simulink library "lib_all"  (Simulink 7.6)
settings: Solver=ode45  SolverMode=SingleTasking  StartTime=0.0  StopTime=10.0  RelTol=1e-3
values from neighbouring files (symbols this model uses):
  map_lib_03 = [6.8679 7.0159 7.4571 8.1849 9.1892 10.456 11.97 13.71 15.655 17.781 20.063 22.474 ... (803 elements, 73x11)]    [scr_simdata.mat]    (also in scr_simdata_all.mat)
  map_lib_06 = [2.5784 2.6727 2.9588 3.4453 4.1444 5.068 6.226 7.6242 9.2634 11.139 13.24 15.552 ... (803 elements, 73x11)]    [scr_simdata.mat]    (also in scr_simdata_all.mat)
  map_lib_09 = [0.0025784 0.0026727 0.0029588 0.0034453 0.0041444 0.005068 0.006226 0.0076242 0.0092634 0.011139 0.01324 0.015552 ... (803 elements, 73x11)]    [scr_simdata.mat]    (also in scr_simdata_all.mat)
  map_lib_10 = [2.74 2.75 2.76 2.77 2.78 2.79 2.8 2.81 2.82 2.83 2.84 2.85 ... (81 elements, 1x81)]    [scr_simdata.mat]    (also in scr_simdata_all.mat)
  map_lib_11 = [1.1983 1.2027 1.207 1.2113 1.2156 1.2198 1.2241 1.2283 1.2326 1.2368 1.241 1.2451 ... (81 elements, 1x81)]    [scr_simdata.mat]    (also in scr_simdata_all.mat)
  map_lib_12 = [2.74 2.75 2.76 2.77 2.78 2.79 2.8 2.81 2.82 2.83 2.84 2.85 ... (81 elements, 1x81)]    [scr_simdata.mat]    (also in scr_simdata_all.mat)
  map_lib_13 = [1.74 1.75 1.76 1.77 1.78 1.79 1.8 1.81 1.82 1.83 1.84 1.85 ... (81 elements, 1x81)]    [scr_simdata.mat]    (also in scr_simdata_all.mat)
  map_lib_16 = [0.00048828125 1.6159 1.8962 2.0821 2.2251 2.3426 2.4433 2.5318 2.611 2.683 2.749 2.8101 ... (1419 elements, 129x11)]    [scr_simdata.mat]    (also in scr_simdata_all.mat)
  map_mr2_01 = [0 800 1600 2400 3200 4000]    [scr_simdata.mat]    (also in scr_simdata_all.mat)
  map_mr2_02 = [1.32 1.32 1.33 1.34 1.36 1.38]    [scr_simdata.mat]    (also in scr_simdata_all.mat)
  map_mr2_03 = [0 800 1600 2400 3200 4000]    [scr_simdata.mat]    (also in scr_simdata_all.mat)
  map_mr2_04 = [1.32 1.32 1.33 1.34 1.36 1.38]    [scr_simdata.mat]    (also in scr_simdata_all.mat)
  map_mr2_05 = [0 800 1600 2400 3200 4000]    [scr_simdata.mat]    (also in scr_simdata_all.mat)
  map_mr2_06 = [1.32 1.32 1.33 1.34 1.36 1.38]    [scr_simdata.mat]    (also in scr_simdata_all.mat)
  Parameter1 = 6    [scr_simdata.mat]    (also in scr_simdata_all.mat)
  Parameter101 = 30    [scr_simdata.mat]    (also in scr_simdata_all.mat)
  Parameter102 = 12    [scr_simdata.mat]    (also in scr_simdata_all.mat)
  Parameter103 = -240    [scr_simdata.mat]    (also in scr_simdata_all.mat)
  Parameter108 = 48    [scr_simdata.mat]    (also in scr_simdata_all.mat)
  Parameter120 = 16    [scr_simdata.mat]    (also in scr_simdata_all.mat)
  Parameter121 = 87    [scr_simdata.mat]    (also in scr_simdata_all.mat)
  Parameter123 = 78    [scr_simdata.mat]    (also in scr_simdata_all.mat)
  Parameter124 = 60    [scr_simdata.mat]    (also in scr_simdata_all.mat)
  Parameter125 = -480    [scr_simdata.mat]    (also in scr_simdata_all.mat)
  Parameter130 = 96    [scr_simdata.mat]    (also in scr_simdata_all.mat)
  Parameter131 = 3    [scr_simdata.mat]    (also in scr_simdata_all.mat)
  Parameter144 = 3    [scr_simdata.mat]    (also in scr_simdata_all.mat)
  Parameter18 = 128    [scr_simdata.mat]    (also in scr_simdata_all.mat)
  Parameter19 = 16    [scr_simdata.mat]    (also in scr_simdata_all.mat)
  Parameter2 = 12    [scr_simdata.mat]    (also in scr_simdata_all.mat)
  Parameter20 = 7    [scr_simdata.mat]    (also in scr_simdata_all.mat)
  Parameter21 = 4    [scr_simdata.mat]    (also in scr_simdata_all.mat)
  Parameter3 = 18    [scr_simdata.mat]    (also in scr_simdata_all.mat)
  Parameter32 = 1    [scr_simdata.mat]    (also in scr_simdata_all.mat)
  Parameter34 = 24    [scr_simdata.mat]    (also in scr_simdata_all.mat)
  Parameter4 = 138    [scr_simdata.mat]    (also in scr_simdata_all.mat)
  Parameter42 = 48    [scr_simdata.mat]    (also in scr_simdata_all.mat)
  Parameter5 = 135    [scr_simdata.mat]    (also in scr_simdata_all.mat)
  Parameter55 = 0    [scr_simdata.mat]    (also in scr_simdata_all.mat)
  Parameter56 = 0    [scr_simdata.mat]    (also in scr_simdata_all.mat)
  Parameter57 = 1    [scr_simdata.mat]    (also in scr_simdata_all.mat)
  Parameter58 = 1    [scr_simdata.mat]    (also in scr_simdata_all.mat)
  Parameter59 = 2    [scr_simdata.mat]    (also in scr_simdata_all.mat)
  Parameter6 = 132    [scr_simdata.mat]    (also in scr_simdata_all.mat)
  Parameter60 = 2    [scr_simdata.mat]    (also in scr_simdata_all.mat)
  Parameter63 = 144    [scr_simdata.mat]    (also in scr_simdata_all.mat)
  Parameter7 = 126    [scr_simdata.mat]    (also in scr_simdata_all.mat)
  Parameter76 = 64    [scr_simdata.mat]    (also in scr_simdata_all.mat)
  Parameter77 = 135    [scr_simdata.mat]    (also in scr_simdata_all.mat)
  Parameter79 = 126    [scr_simdata.mat]    (also in scr_simdata_all.mat)
  Parameter80 = 108    [scr_simdata.mat]    (also in scr_simdata_all.mat)
  Parameter81 = 0    [scr_simdata.mat]    (also in scr_simdata_all.mat)
  Parameter86 = 0    [scr_simdata.mat]    (also in scr_simdata_all.mat)
  Parameter98 = 112    [scr_simdata.mat]    (also in scr_simdata_all.mat)
  Parameter99 = 39    [scr_simdata.mat]    (also in scr_simdata_all.mat)
undefined symbols (not defined in the file or in its folder): cylvolkp_hih_map2h, cylvolkp_map2h, pk1k_map2h
libraries: simulink x2
(part 19 of 36)

== system lib_all/SubSystem1593/SubSystem1648/SubSystem1650/SubSystem1651/SubSystem1653 ==
  note: ***編集禁止***
  In1 : Inport
  In2 : Inport  Port=2
  RO1 : RelationalOperator  Operator===  InputSameDT=on
  single1 : DataTypeConversion  OutDataTypeStr=single  SaturateOnIntegerOverflow=on  RndMeth=Zero
  single2 : DataTypeConversion  OutDataTypeStr=single  SaturateOnIntegerOverflow=on  RndMeth=Zero
  Out1 : Outport
  wires:
  RO1/1 -> Out1/1
  In2/1 -> single2/1
  In1/1 -> single1/1
  single1/1 -> RO1/1
  single2/1 -> RO1/2

== system lib_all/SubSystem1593/SubSystem1648/SubSystem1650/SubSystem1651/SubSystem1654 ==
  Block4415 : Inport
  Block4416 : Inport  Port=2
  Block4417 : Inport  Port=3
  Block4418 : Sum  OutDataTypeStr=double  RndMeth=Zero  SaturateOnIntegerOverflow=on  InputSameDT=on
  Block4419 : Switch  Threshold=0.5  RndMeth=Zero  SaturateOnIntegerOverflow=on  InputSameDT=on
  SubSystem1655 : Subsystem
  Block4420 : Outport
  wires:
  Block4415/1 -> Block4418/1
  Block4419/1 -> Block4420/1
  Block4418/1 -> Block4419/1
  SubSystem1655/1(Out1) -> Block4419/2
  Block4417/1 -> SubSystem1655/2(In2)
  Block4416/1 -> Block4418/2, Block4419/3, SubSystem1655/1(In1)

== system lib_all/SubSystem1593/SubSystem1648/SubSystem1650/SubSystem1656/SubSystem1657 ==
  note: ***編集禁止***
  In1 : Inport
  In2 : Inport  Port=2
  LO1 : Logic
  RO1 : RelationalOperator  Operator=<  InputSameDT=on
  RO2 : RelationalOperator  Operator=~=  InputSameDT=on
  single1 : DataTypeConversion  OutDataTypeStr=single  SaturateOnIntegerOverflow=on  RndMeth=Zero
  single2 : DataTypeConversion  OutDataTypeStr=single  SaturateOnIntegerOverflow=on  RndMeth=Zero
  Out1 : Outport
  wires:
  In2/1 -> single2/1
  In1/1 -> single1/1
  single1/1 -> RO1/1, RO2/1
  single2/1 -> RO1/2, RO2/2
  RO1/1 -> LO1/1
  RO2/1 -> LO1/2
  LO1/1 -> Out1/1

== system lib_all/SubSystem1662/SubSystem1664/SubSystem1665/SubSystem1668/SubSystem1669 ==
  note: ***編集禁止***
  In1 : Inport
  In2 : Inport  Port=2
  LO1 : Logic
  RO1 : RelationalOperator  Operator=<  InputSameDT=on
  RO2 : RelationalOperator  Operator=~=  InputSameDT=on
  single1 : DataTypeConversion  OutDataTypeStr=single  SaturateOnIntegerOverflow=on  RndMeth=Zero
  single2 : DataTypeConversion  OutDataTypeStr=single  SaturateOnIntegerOverflow=on  RndMeth=Zero
  Out1 : Outport
  wires:
  In2/1 -> single2/1
  In1/1 -> single1/1
  single1/1 -> RO1/1, RO2/1
  single2/1 -> RO1/2, RO2/2
  RO1/1 -> LO1/1
  RO2/1 -> LO1/2
  LO1/1 -> Out1/1

== system lib_all/SubSystem1674/SubSystem1687/SubSystem1690/SubSystem1691/SubSystem1692 ==
  note: ***編集禁止***
  In1 : Inport
  In2 : Inport  Port=2
  RO1 : RelationalOperator  Operator===  InputSameDT=on
  single1 : DataTypeConversion  OutDataTypeStr=single  SaturateOnIntegerOverflow=on  RndMeth=Zero
  single2 : DataTypeConversion  OutDataTypeStr=single  SaturateOnIntegerOverflow=on  RndMeth=Zero
  Out1 : Outport
  wires:
  single2/1 -> RO1/2
  single1/1 -> RO1/1
  In1/1 -> single1/1
  In2/1 -> single2/1
  RO1/1 -> Out1/1

== system lib_all/SubSystem1674/SubSystem1687/SubSystem1690/SubSystem1691/SubSystem1693 ==
  note: ***編集禁止***
  In1 : Inport
  In2 : Inport  Port=2
  RO1 : RelationalOperator  Operator===  InputSameDT=on
  single1 : DataTypeConversion  OutDataTypeStr=single  SaturateOnIntegerOverflow=on  RndMeth=Zero
  single2 : DataTypeConversion  OutDataTypeStr=single  SaturateOnIntegerOverflow=on  RndMeth=Zero
  Out1 : Outport
  wires:
  single2/1 -> RO1/2
  single1/1 -> RO1/1
  In1/1 -> single1/1
  In2/1 -> single2/1
  RO1/1 -> Out1/1

== system lib_all/SubSystem1674/SubSystem1687/SubSystem1690/SubSystem1691/SubSystem1694 ==
  Block4594 : Inport
  Block4595 : Inport  Port=2
  Block4596 : Inport  Port=3
  Block4597 : Sum  OutDataTypeStr=double  RndMeth=Zero  SaturateOnIntegerOverflow=on  InputSameDT=on
  Block4598 : Switch  Threshold=0.5  RndMeth=Zero  SaturateOnIntegerOverflow=on  InputSameDT=on
  SubSystem1695 : Subsystem
  Block4599 : Outport
  wires:
  Block4595/1 -> SubSystem1695/1(In1), Block4598/3, Block4597/2
  Block4596/1 -> SubSystem1695/2(In2)
  SubSystem1695/1(Out1) -> Block4598/2
  Block4597/1 -> Block4598/1
  Block4598/1 -> Block4599/1
  Block4594/1 -> Block4597/1

== system lib_all/SubSystem1674/SubSystem1687/SubSystem1690/SubSystem1696/SubSystem1697 ==
  note: ***編集禁止***
  In1 : Inport
  In2 : Inport  Port=2
  LO1 : Logic
  RO1 : RelationalOperator  Operator=<  InputSameDT=on
  RO2 : RelationalOperator  Operator=~=  InputSameDT=on
  single1 : DataTypeConversion  OutDataTypeStr=single  SaturateOnIntegerOverflow=on  RndMeth=Zero
  single2 : DataTypeConversion  OutDataTypeStr=single  SaturateOnIntegerOverflow=on  RndMeth=Zero
  Out1 : Outport
  wires:
  LO1/1 -> Out1/1
  RO2/1 -> LO1/2
  RO1/1 -> LO1/1
  single2/1 -> RO2/2, RO1/2
  single1/1 -> RO2/1, RO1/1
  In1/1 -> single1/1
  In2/1 -> single2/1

== system lib_all/SubSystem1674/SubSystem1701/SubSystem1703/SubSystem1704/Product ==
  note: ***編集禁止***
  mult : Inport
  div : Inport  Port=2
  init : Inport  Port=3
  Constant1 : Constant  OutDataTypeStr=Inherit: Inherit via back propagation
  Constant2 : Constant  Value=0  OutDataTypeStr=Inherit: Inherit via back propagation
  Product : Product  Inputs=*/  SaturateOnIntegerOverflow=on  RndMeth=Zero  InputSameDT=on  OutDataTypeStr=Inherit: Same as first input
  Relational Operator : RelationalOperator  Operator=~=  InputSameDT=on
  Switch : Switch  Threshold=0.5  SaturateOnIntegerOverflow=on  InputSameDT=on
  Switch1 : Switch  Threshold=0.5  SaturateOnIntegerOverflow=on  InputSameDT=on
  Out : Outport
  wires:
  mult/1 -> Product/1
  Product/1 -> Switch/1
  Relational Operator/1 -> Switch/2, Switch1/2
  init/1 -> Switch/3
  Switch/1 -> Out/1
  div/1 -> Switch1/1, Relational Operator/1
  Switch1/1 -> Product/2
  Constant2/1 -> Relational Operator/2
  Constant1/1 -> Switch1/3

== system lib_all/SubSystem1674/SubSystem1701/SubSystem1703/SubSystem1706/SubSystem1707 ==
  note: ***編集禁止***
  In1 : Inport
  In2 : Inport  Port=2
  RO1 : RelationalOperator  Operator=>  InputSameDT=on
  single1 : DataTypeConversion  OutDataTypeStr=single  SaturateOnIntegerOverflow=on  RndMeth=Zero
  single2 : DataTypeConversion  OutDataTypeStr=single  SaturateOnIntegerOverflow=on  RndMeth=Zero
  Out1 : Outport
  wires:
  single2/1 -> RO1/2
  single1/1 -> RO1/1
  In1/1 -> single1/1
  In2/1 -> single2/1
  RO1/1 -> Out1/1

== system lib_all/SubSystem1674/SubSystem1701/SubSystem1703/SubSystem1706/SubSystem1708 ==
  note: ***編集禁止***
  In1 : Inport
  In2 : Inport  Port=2
  LO1 : Logic
  RO1 : RelationalOperator  Operator=<  InputSameDT=on
  RO2 : RelationalOperator  Operator=~=  InputSameDT=on
  single1 : DataTypeConversion  OutDataTypeStr=single  SaturateOnIntegerOverflow=on  RndMeth=Zero
  single2 : DataTypeConversion  OutDataTypeStr=single  SaturateOnIntegerOverflow=on  RndMeth=Zero
  Out1 : Outport
  wires:
  LO1/1 -> Out1/1
  RO2/1 -> LO1/2
  RO1/1 -> LO1/1
  single2/1 -> RO2/2, RO1/2
  single1/1 -> RO2/1, RO1/1
  In1/1 -> single1/1
  In2/1 -> single2/1

== system lib_all/SubSystem1674/SubSystem1701/SubSystem1709/SubSystem1713/SubSystem1714 ==
  Block4682 : Inport
  Block4683 : Inport  Port=2
  Block4684 : MultiPortSwitch  zeroidx=off  RndMeth=Zero  SaturateOnIntegerOverflow=on  InputSameDT=on
  SubSystem1715 : Subsystem  TreatAsAtomicUnit=on  mask(***ATC.DSRVEC***){bd_version=1.01.A, r0=DSM18, r1=-1, r2=, r3=, r4=, r5=, r6=, r7=, r8=, r9=, c0=, c1=, c2=, c3=, c4=, c5=, c6=, c7=, c8=, c9=}
      mask prompts: bd_version "Version"
      mask help: これはAutoCからのブロックです．編集禁止．
      settings: OpenFcn=warndlg('変更禁止!');
      mask Help: web(ui_blockhelpmng(gcb));
      mask Display: disp('ATC.DSRVEC')
  SubSystem1716 : Subsystem  TreatAsAtomicUnit=on  mask(***ATC.DSRVEC***){bd_version=1.01.A, r0=DSM8, r1=-1, r2=, r3=, r4=, r5=, r6=, r7=, r8=, r9=, c0=, c1=, c2=, c3=, c4=, c5=, c6=, c7=, c8=, c9=}
      mask prompts: bd_version "Version"
      mask help: これはAutoCからのブロックです．編集禁止．
      settings: OpenFcn=warndlg('変更禁止!');
      mask Help: web(ui_blockhelpmng(gcb));
      mask Display: disp('ATC.DSRVEC')
  SubSystem1717 : Subsystem  TreatAsAtomicUnit=on  mask(***ATC.DSRVEC***){bd_version=1.01.A, r0=DSM11, r1=-1, r2=, r3=, r4=, r5=, r6=, r7=, r8=, r9=, c0=, c1=, c2=, c3=, c4=, c5=, c6=, c7=, c8=, c9=}
      mask prompts: bd_version "Version"
      mask help: これはAutoCからのブロックです．編集禁止．
      settings: OpenFcn=warndlg('変更禁止!');
      mask Help: web(ui_blockhelpmng(gcb));
      mask Display: disp('ATC.DSRVEC')
  Block4685 : Outport
  wires:
  Block4683/1 -> SubSystem1717/1(element), SubSystem1716/1(element), SubSystem1715/1(element)
  SubSystem1717/1(Output) -> Block4684/4
  SubSystem1716/1(Output) -> Block4684/3
  SubSystem1715/1(Output) -> Block4684/2
  Block4684/1 -> Block4685/1
  Block4682/1 -> Block4684/1

== system lib_all/SubSystem1674/SubSystem1701/SubSystem1709/SubSystem1713/SubSystem1718 ==
  Block4686 : Inport
  Block4687 : Inport  Port=2
  Block4688 : MultiPortSwitch  zeroidx=off  RndMeth=Zero  SaturateOnIntegerOverflow=on  InputSameDT=on
  SubSystem1719 : Subsystem  TreatAsAtomicUnit=on  mask(***ATC.DSRVEC***){bd_version=1.01.A, r0=DSM18, r1=-1, r2=, r3=, r4=, r5=, r6=, r7=, r8=, r9=, c0=, c1=, c2=, c3=, c4=, c5=, c6=, c7=, c8=, c9=}
      mask prompts: bd_version "Version"
      mask help: これはAutoCからのブロックです．編集禁止．
      settings: OpenFcn=warndlg('変更禁止!');
      mask Help: web(ui_blockhelpmng(gcb));
      mask Display: disp('ATC.DSRVEC')
  SubSystem1720 : Subsystem  TreatAsAtomicUnit=on  mask(***ATC.DSRVEC***){bd_version=1.01.A, r0=DSM8, r1=-1, r2=, r3=, r4=, r5=, r6=, r7=, r8=, r9=, c0=, c1=, c2=, c3=, c4=, c5=, c6=, c7=, c8=, c9=}
      mask prompts: bd_version "Version"
      mask help: これはAutoCからのブロックです．編集禁止．
      settings: OpenFcn=warndlg('変更禁止!');
      mask Help: web(ui_blockhelpmng(gcb));
      mask Display: disp('ATC.DSRVEC')
  SubSystem1721 : Subsystem  TreatAsAtomicUnit=on  mask(***ATC.DSRVEC***){bd_version=1.01.A, r0=DSM11, r1=-1, r2=, r3=, r4=, r5=, r6=, r7=, r8=, r9=, c0=, c1=, c2=, c3=, c4=, c5=, c6=, c7=, c8=, c9=}
      mask prompts: bd_version "Version"
      mask help: これはAutoCからのブロックです．編集禁止．
      settings: OpenFcn=warndlg('変更禁止!');
      mask Help: web(ui_blockhelpmng(gcb));
      mask Display: disp('ATC.DSRVEC')
  Block4689 : Outport
  wires:
  Block4687/1 -> SubSystem1721/1(element), SubSystem1720/1(element), SubSystem1719/1(element)
  SubSystem1721/1(Output) -> Block4688/4
  SubSystem1720/1(Output) -> Block4688/3
  SubSystem1719/1(Output) -> Block4688/2
  Block4688/1 -> Block4689/1
  Block4686/1 -> Block4688/1

== system lib_all/SubSystem1674/SubSystem1701/SubSystem1709/SubSystem1722/SubSystem1723 ==
  note: ***編集禁止***
  element : Inport
  Inside_DSR : DataStoreRead  DataStoreName=DSM4
  Selector : Selector  IndexOptions=Index vector (port)  Indices=[1 3]  OutputSizes=1
  Output : Outport
  wires:
  Inside_DSR/1 -> Selector/1
  element/1 -> Selector/2
  Selector/1 -> Output/1

== system lib_all/SubSystem1674/SubSystem1701/SubSystem1709/SubSystem1722/SubSystem1724 ==
  Block4697 : Inport
  Block4698 : Inport  Port=2
  Block4699 : MultiPortSwitch  zeroidx=off  RndMeth=Zero  SaturateOnIntegerOverflow=on  InputSameDT=on
  SubSystem1725 : Subsystem  TreatAsAtomicUnit=on  mask(***ATC.DSRVEC***){bd_version=1.01.A, r0=DSM18, r1=-1, r2=, r3=, r4=, r5=, r6=, r7=, r8=, r9=, c0=, c1=, c2=, c3=, c4=, c5=, c6=, c7=, c8=, c9=}
      mask prompts: bd_version "Version"
      mask help: これはAutoCからのブロックです．編集禁止．
      settings: OpenFcn=warndlg('変更禁止!');
      mask Help: web(ui_blockhelpmng(gcb));
      mask Display: disp('ATC.DSRVEC')
  SubSystem1726 : Subsystem  TreatAsAtomicUnit=on  mask(***ATC.DSRVEC***){bd_version=1.01.A, r0=DSM8, r1=-1, r2=, r3=, r4=, r5=, r6=, r7=, r8=, r9=, c0=, c1=, c2=, c3=, c4=, c5=, c6=, c7=, c8=, c9=}
      mask prompts: bd_version "Version"
      mask help: これはAutoCからのブロックです．編集禁止．
      settings: OpenFcn=warndlg('変更禁止!');
      mask Help: web(ui_blockhelpmng(gcb));
      mask Display: disp('ATC.DSRVEC')
  SubSystem1727 : Subsystem  TreatAsAtomicUnit=on  mask(***ATC.DSRVEC***){bd_version=1.01.A, r0=DSM11, r1=-1, r2=, r3=, r4=, r5=, r6=, r7=, r8=, r9=, c0=, c1=, c2=, c3=, c4=, c5=, c6=, c7=, c8=, c9=}
      mask prompts: bd_version "Version"
      mask help: これはAutoCからのブロックです．編集禁止．
      settings: OpenFcn=warndlg('変更禁止!');
      mask Help: web(ui_blockhelpmng(gcb));
      mask Display: disp('ATC.DSRVEC')
  Block4700 : Outport
  wires:
  Block4698/1 -> SubSystem1727/1(element), SubSystem1726/1(element), SubSystem1725/1(element)
  SubSystem1727/1(Output) -> Block4699/4
  SubSystem1726/1(Output) -> Block4699/3
  SubSystem1725/1(Output) -> Block4699/2
  Block4699/1 -> Block4700/1
  Block4697/1 -> Block4699/1

== system lib_all/SubSystem1674/SubSystem1701/SubSystem1709/SubSystem1722/SubSystem1728 ==
  Block4701 : Inport
  Block4702 : Inport  Port=2
  Block4703 : MultiPortSwitch  zeroidx=off  RndMeth=Zero  SaturateOnIntegerOverflow=on  InputSameDT=on
  SubSystem1729 : Subsystem  TreatAsAtomicUnit=on  mask(***ATC.DSRVEC***){bd_version=1.01.A, r0=DSM18, r1=-1, r2=, r3=, r4=, r5=, r6=, r7=, r8=, r9=, c0=, c1=, c2=, c3=, c4=, c5=, c6=, c7=, c8=, c9=}
      mask prompts: bd_version "Version"
      mask help: これはAutoCからのブロックです．編集禁止．
      settings: OpenFcn=warndlg('変更禁止!');
      mask Help: web(ui_blockhelpmng(gcb));
      mask Display: disp('ATC.DSRVEC')
  SubSystem1730 : Subsystem  TreatAsAtomicUnit=on  mask(***ATC.DSRVEC***){bd_version=1.01.A, r0=DSM8, r1=-1, r2=, r3=, r4=, r5=, r6=, r7=, r8=, r9=, c0=, c1=, c2=, c3=, c4=, c5=, c6=, c7=, c8=, c9=}
      mask prompts: bd_version "Version"
      mask help: これはAutoCからのブロックです．編集禁止．
      settings: OpenFcn=warndlg('変更禁止!');
      mask Help: web(ui_blockhelpmng(gcb));
      mask Display: disp('ATC.DSRVEC')
  SubSystem1731 : Subsystem  TreatAsAtomicUnit=on  mask(***ATC.DSRVEC***){bd_version=1.01.A, r0=DSM11, r1=-1, r2=, r3=, r4=, r5=, r6=, r7=, r8=, r9=, c0=, c1=, c2=, c3=, c4=, c5=, c6=, c7=, c8=, c9=}
      mask prompts: bd_version "Version"
      mask help: これはAutoCからのブロックです．編集禁止．
      settings: OpenFcn=warndlg('変更禁止!');
      mask Help: web(ui_blockhelpmng(gcb));
      mask Display: disp('ATC.DSRVEC')
  Block4704 : Outport
  wires:
  Block4702/1 -> SubSystem1731/1(element), SubSystem1730/1(element), SubSystem1729/1(element)
  SubSystem1731/1(Output) -> Block4703/4
  SubSystem1730/1(Output) -> Block4703/3
  SubSystem1729/1(Output) -> Block4703/2
  Block4703/1 -> Block4704/1
  Block4701/1 -> Block4703/1

== system lib_all/SubSystem1674/SubSystem1701/SubSystem1709/SubSystem1732/SubSystem1733 ==
  note: ***編集禁止***
  element : Inport
  Inside_DSR : DataStoreRead  DataStoreName=DSM2
  Selector : Selector  IndexOptions=Index vector (port)  Indices=[1 3]  OutputSizes=1
  Output : Outport
  wires:
  Inside_DSR/1 -> Selector/1
  element/1 -> Selector/2
  Selector/1 -> Output/1

== system lib_all/SubSystem1674/SubSystem1701/SubSystem1709/SubSystem1732/SubSystem1734 ==
  Block4712 : Inport
  Block4713 : Inport  Port=2
  Block4714 : MultiPortSwitch  zeroidx=off  RndMeth=Zero  SaturateOnIntegerOverflow=on  InputSameDT=on
  SubSystem1735 : Subsystem  TreatAsAtomicUnit=on  mask(***ATC.DSRVEC***){bd_version=1.01.A, r0=DSM18, r1=-1, r2=, r3=, r4=, r5=, r6=, r7=, r8=, r9=, c0=, c1=, c2=, c3=, c4=, c5=, c6=, c7=, c8=, c9=}
      mask prompts: bd_version "Version"
      mask help: これはAutoCからのブロックです．編集禁止．
      settings: OpenFcn=warndlg('変更禁止!');
      mask Help: web(ui_blockhelpmng(gcb));
      mask Display: disp('ATC.DSRVEC')
  SubSystem1736 : Subsystem  TreatAsAtomicUnit=on  mask(***ATC.DSRVEC***){bd_version=1.01.A, r0=DSM8, r1=-1, r2=, r3=, r4=, r5=, r6=, r7=, r8=, r9=, c0=, c1=, c2=, c3=, c4=, c5=, c6=, c7=, c8=, c9=}
      mask prompts: bd_version "Version"
      mask help: これはAutoCからのブロックです．編集禁止．
      settings: OpenFcn=warndlg('変更禁止!');
      mask Help: web(ui_blockhelpmng(gcb));
      mask Display: disp('ATC.DSRVEC')
  SubSystem1737 : Subsystem  TreatAsAtomicUnit=on  mask(***ATC.DSRVEC***){bd_version=1.01.A, r0=DSM11, r1=-1, r2=, r3=, r4=, r5=, r6=, r7=, r8=, r9=, c0=, c1=, c2=, c3=, c4=, c5=, c6=, c7=, c8=, c9=}
      mask prompts: bd_version "Version"
      mask help: これはAutoCからのブロックです．編集禁止．
      settings: OpenFcn=warndlg('変更禁止!');
      mask Help: web(ui_blockhelpmng(gcb));
      mask Display: disp('ATC.DSRVEC')
  Block4715 : Outport
  wires:
  Block4713/1 -> SubSystem1737/1(element), SubSystem1736/1(element), SubSystem1735/1(element)
  SubSystem1737/1(Output) -> Block4714/4
  SubSystem1736/1(Output) -> Block4714/3
  SubSystem1735/1(Output) -> Block4714/2
  Block4714/1 -> Block4715/1
  Block4712/1 -> Block4714/1

== system lib_all/SubSystem1674/SubSystem1701/SubSystem1709/SubSystem1732/SubSystem1738 ==
  Block4716 : Inport
  Block4717 : Inport  Port=2
  Block4718 : MultiPortSwitch  zeroidx=off  RndMeth=Zero  SaturateOnIntegerOverflow=on  InputSameDT=on
  SubSystem1739 : Subsystem  TreatAsAtomicUnit=on  mask(***ATC.DSRVEC***){bd_version=1.01.A, r0=DSM18, r1=-1, r2=, r3=, r4=, r5=, r6=, r7=, r8=, r9=, c0=, c1=, c2=, c3=, c4=, c5=, c6=, c7=, c8=, c9=}
      mask prompts: bd_version "Version"
      mask help: これはAutoCからのブロックです．編集禁止．
      settings: OpenFcn=warndlg('変更禁止!');
      mask Help: web(ui_blockhelpmng(gcb));
      mask Display: disp('ATC.DSRVEC')
  SubSystem1740 : Subsystem  TreatAsAtomicUnit=on  mask(***ATC.DSRVEC***){bd_version=1.01.A, r0=DSM8, r1=-1, r2=, r3=, r4=, r5=, r6=, r7=, r8=, r9=, c0=, c1=, c2=, c3=, c4=, c5=, c6=, c7=, c8=, c9=}
      mask prompts: bd_version "Version"
      mask help: これはAutoCからのブロックです．編集禁止．
      settings: OpenFcn=warndlg('変更禁止!');
      mask Help: web(ui_blockhelpmng(gcb));
      mask Display: disp('ATC.DSRVEC')
  SubSystem1741 : Subsystem  TreatAsAtomicUnit=on  mask(***ATC.DSRVEC***){bd_version=1.01.A, r0=DSM11, r1=-1, r2=, r3=, r4=, r5=, r6=, r7=, r8=, r9=, c0=, c1=, c2=, c3=, c4=, c5=, c6=, c7=, c8=, c9=}
      mask prompts: bd_version "Version"
      mask help: これはAutoCからのブロックです．編集禁止．
      settings: OpenFcn=warndlg('変更禁止!');
      mask Help: web(ui_blockhelpmng(gcb));
      mask Display: disp('ATC.DSRVEC')
  Block4719 : Outport
  wires:
  Block4717/1 -> SubSystem1741/1(element), SubSystem1740/1(element), SubSystem1739/1(element)
  SubSystem1741/1(Output) -> Block4718/4
  SubSystem1740/1(Output) -> Block4718/3
  SubSystem1739/1(Output) -> Block4718/2
  Block4718/1 -> Block4719/1
  Block4716/1 -> Block4718/1

== system lib_all/SubSystem1742/SubSystem1745/SubSystem1746/SubSystem1749/SubSystem1750 ==
  note: ***編集禁止***
  In1 : Inport
  In2 : Inport  Port=2
  LO1 : Logic
  RO1 : RelationalOperator  Operator=<  InputSameDT=on
  RO2 : RelationalOperator  Operator=~=  InputSameDT=on
  single1 : DataTypeConversion  OutDataTypeStr=single  SaturateOnIntegerOverflow=on  RndMeth=Zero
  single2 : DataTypeConversion  OutDataTypeStr=single  SaturateOnIntegerOverflow=on  RndMeth=Zero
  Out1 : Outport
  wires:
  In2/1 -> single2/1
  In1/1 -> single1/1
  single1/1 -> RO1/1, RO2/1
  single2/1 -> RO1/2, RO2/2
  RO1/1 -> LO1/1
  RO2/1 -> LO1/2
  LO1/1 -> Out1/1

== system lib_all/SubSystem1756/SubSystem1759/SubSystem1760/SubSystem1763/SubSystem1764 ==
  note: ***編集禁止***
  In1 : Inport
  In2 : Inport  Port=2
  LO1 : Logic
  RO1 : RelationalOperator  Operator=<  InputSameDT=on
  RO2 : RelationalOperator  Operator=~=  InputSameDT=on
  single1 : DataTypeConversion  OutDataTypeStr=single  SaturateOnIntegerOverflow=on  RndMeth=Zero
  single2 : DataTypeConversion  OutDataTypeStr=single  SaturateOnIntegerOverflow=on  RndMeth=Zero
  Out1 : Outport
  wires:
  LO1/1 -> Out1/1
  RO2/1 -> LO1/2
  RO1/1 -> LO1/1
  single2/1 -> RO2/2, RO1/2
  single1/1 -> RO2/1, RO1/1
  In1/1 -> single1/1
  In2/1 -> single2/1

== system lib_all/SubSystem176/SubSystem178/SubSystem182/SubSystem183/SubSystem185 ==
  note: ***編集禁止***
  In1 : Inport
  In2 : Inport  Port=2
  LO1 : Logic
  RO1 : RelationalOperator  Operator=<  InputSameDT=on
  RO2 : RelationalOperator  Operator=~=  InputSameDT=on
  single1 : DataTypeConversion  OutDataTypeStr=single  SaturateOnIntegerOverflow=on  RndMeth=Zero
  single2 : DataTypeConversion  OutDataTypeStr=single  SaturateOnIntegerOverflow=on  RndMeth=Zero
  Out1 : Outport
  wires:
  In2/1 -> single2/1
  In1/1 -> single1/1
  single1/1 -> RO1/1, RO2/1
  single2/1 -> RO1/2, RO2/2
  RO1/1 -> LO1/1
  RO2/1 -> LO1/2
  LO1/1 -> Out1/1

== system lib_all/SubSystem176/SubSystem178/SubSystem182/SubSystem183/SubSystem186 ==
  note: Text31
  note: Text30
  Block426 : Inport
  Block427 : Inport  Port=2
  Block428 : Inport  Port=3
  Block429 : Constant  Value=720  OutDataTypeStr=double  SampleTime=-1
  Block430 : Constant  Value=0  OutDataTypeStr=double  SampleTime=-1
  Block431 : Constant  Value=0  OutDataTypeStr=double  SampleTime=-1
  Block432 : Constant  Value=5  OutDataTypeStr=double  SampleTime=-1
  Block433 : DataStoreRead  DataStoreName=DSM50
  Block434 : DataStoreRead  DataStoreName=DSM35
  Block435 : Gain  Gain=5  SaturateOnIntegerOverflow=on  OutDataTypeStr=Inherit: Same as input  ParamDataTypeStr=Inherit: Same as input
      settings: AttributesFormatString=Attribute105
  Block436 : Gain  Gain=5  SaturateOnIntegerOverflow=on  OutDataTypeStr=Inherit: Same as input  ParamDataTypeStr=Inherit: Same as input
      settings: AttributesFormatString=Attribute106
  Block437 : Gain  Gain=1/5  SaturateOnIntegerOverflow=on  OutDataTypeStr=Inherit: Same as input  ParamDataTypeStr=Inherit: Same as input
      settings: AttributesFormatString=Attribute107
  Block438 : Product  Inputs=*/  OutDataTypeStr=double  SaturateOnIntegerOverflow=on  RndMeth=Zero  InputSameDT=on
  Block439 : Rounding
  Block440 : Rounding
  Block441 : Sum  Inputs=+-+-  OutDataTypeStr=double  RndMeth=Zero  SaturateOnIntegerOverflow=on  InputSameDT=on
  Block442 : Sum  Inputs=-+  OutDataTypeStr=double  RndMeth=Zero  SaturateOnIntegerOverflow=on  InputSameDT=on
  SubSystem187 : Subsystem  TreatAsAtomicUnit=on  mask(***ATC.DIVISION***){bd_version=1.01.A, r0=チェック有り, r1=, r2=, r3=, r4=, r5=, r6=, r7=, r8=, r9=, c0=, c1=, c2=, c3=, c4=, c5=, c6=, c7=, c8=, c9=}
      mask prompts: bd_version "Version"
      mask help: これはAutoCからのブロックです．編集禁止．
      settings: OpenFcn=warndlg('変更禁止!');
      mask Help: web(ui_blockhelpmng(gcb));
  SubSystem188 : Subsystem  TreatAsAtomicUnit=on  mask(***ATC.DSRVEC***){bd_version=1.01.A, r0=DSM95, r1=-1, r2=, r3=, r4=, r5=, r6=, r7=, r8=, r9=, c0=, c1=, c2=, c3=, c4=, c5=, c6=, c7=, c8=, c9=}
      mask prompts: bd_version "Version"
      mask help: これはAutoCからのブロックです．編集禁止．
      settings: OpenFcn=warndlg('変更禁止!');
      mask Help: web(ui_blockhelpmng(gcb));
      mask Display: disp('ATC.DSRVEC')
  SubSystem189 : Subsystem
      settings: RTWSystemCode=Inline
  Block451 : Outport
  Block452 : Outport  Port=2
  wires:
  Block440/1 -> Block451/1
  Block437/1 -> Block440/1
  Block436/1 -> Block441/1
  Block433/1 -> Block441/2
  SubSystem188/1(Output) -> SubSystem187/1(mult)
  Block430/1 -> SubSystem187/3(init)
  SubSystem187/1(Out) -> Block441/3  "SigLabel10"
  Block426/1 -> Block436/1
  SubSystem189/1(Block450) -> Block437/1, Block442/2, Block438/1
  Block428/1 -> Block441/4
  Block429/1 -> SubSystem189/1(Block443)
  Block441/1 -> SubSystem189/2(Block444)  "SigLabel9"
  Block431/1 -> SubSystem189/3(Block445)
  Block427/1 -> SubSystem188/1(element)
  Block432/1 -> Block438/2
  Block438/1 -> Block439/1
  Block439/1 -> Block435/1
  Block435/1 -> Block442/1
  Block442/1 -> Block452/1
  Block434/1 -> SubSystem187/2(div)

== system lib_all/SubSystem176/SubSystem178/SubSystem182/SubSystem183/SubSystem192 ==
  note: Text34
  note: Text33
  note: Text32
  Block453 : Inport
  Block454 : Inport  Port=2
  Block455 : Inport  Port=3
  Block456 : Constant  Value=0  OutDataTypeStr=double  SampleTime=-1
  Block457 : Constant  OutDataTypeStr=double  SampleTime=-1
  Block458 : Constant  OutDataTypeStr=double  SampleTime=-1
  Block459 : Constant  Value=0  OutDataTypeStr=double  SampleTime=-1
  Block460 : Constant  Value=2  OutDataTypeStr=double  SampleTime=-1
  Block461 : Constant  OutDataTypeStr=double  SampleTime=-1
  Block462 : Constant  Value=143  OutDataTypeStr=double  SampleTime=-1
  Block463 : Demux  Outputs=2
  Block464 : Logic  Operator=NOT
  Block465 : Mux  Inputs=2
  Block466 : Mux  Inputs=2
  Block467 : Sum  OutDataTypeStr=double  RndMeth=Zero  SaturateOnIntegerOverflow=on  InputSameDT=on
  Block468 : Sum  OutDataTypeStr=double  RndMeth=Zero  SaturateOnIntegerOverflow=on  InputSameDT=on
  Block{469..472} (4 alike) : Switch  Threshold=0.5  RndMeth=Zero  SaturateOnIntegerOverflow=on  InputSameDT=on
  SubSystem193 : Subsystem
  SubSystem194 : Subsystem
  SubSystem195 : Subsystem
  SubSystem196 : Subsystem
  SubSystem197 : Subsystem  TreatAsAtomicUnit=on
  SubSystem206 : Subsystem  TreatAsAtomicUnit=on
  SubSystem216 : Subsystem  TreatAsAtomicUnit=on
  SubSystem226 : Subsystem  TreatAsAtomicUnit=on
  Block532 : Outport
  Block533 : Outport  Port=2
  wires:
  Block471/1 -> Block468/2
  Block461/1 -> Block468/1
  Block453/1 -> SubSystem193/1(In1), Block467/2, Block465/1
  Block462/1 -> SubSystem193/2(In2), Block466/1
  Block454/1 -> SubSystem194/1(In1), SubSystem195/1(In1), SubSystem196/1(In1)
  Block456/1 -> SubSystem194/2(In2)
  SubSystem194/1(Out1) -> Block469/2, SubSystem197/Enable
  SubSystem197/1(Block486) -> Block469/1
  Block457/1 -> SubSystem195/2(In2)
  SubSystem195/1(Out1) -> Block470/2, SubSystem206/Enable
  SubSystem206/1(Block501) -> Block470/1
  Block470/1 -> Block469/3
  Block468/1 -> SubSystem197/1(Block473), SubSystem206/1(Block487), SubSystem216/1(Block502), SubSystem226/1(Block517)
  Block463/1 -> Block532/1
  Block463/2 -> Block533/1
  Block469/1 -> Block463/1
  SubSystem193/1(Out1) -> Block471/2
  Block458/1 -> Block467/1
  Block459/1 -> Block466/2
  Block466/1 -> Block471/3
  Block467/1 -> Block465/2
  Block465/1 -> Block471/1
  Block460/1 -> SubSystem196/2(In2)
  SubSystem196/1(Out1) -> Block464/1, SubSystem216/Enable, Block472/2
  Block464/1 -> SubSystem226/Enable
  SubSystem216/1(Block516) -> Block472/1
  SubSystem226/1(Block531) -> Block472/3
  Block472/1 -> Block470/3
  Block455/1 -> SubSystem197/2(Block474), SubSystem206/2(Block488), SubSystem216/2(Block503), SubSystem226/2(Block518)

== system lib_all/SubSystem176/SubSystem178/SubSystem182/SubSystem236/SubSystem238 ==
  note: ***編集禁止***
  In1 : Inport
  In2 : Inport  Port=2
  LO1 : Logic
  RO1 : RelationalOperator  Operator=<  InputSameDT=on
  RO2 : RelationalOperator  Operator=~=  InputSameDT=on
  single1 : DataTypeConversion  OutDataTypeStr=single  SaturateOnIntegerOverflow=on  RndMeth=Zero
  single2 : DataTypeConversion  OutDataTypeStr=single  SaturateOnIntegerOverflow=on  RndMeth=Zero
  Out1 : Outport
  wires:
  In2/1 -> single2/1
  In1/1 -> single1/1
  single1/1 -> RO1/1, RO2/1
  single2/1 -> RO1/2, RO2/2
  RO1/1 -> LO1/1
  RO2/1 -> LO1/2
  LO1/1 -> Out1/1

== system lib_all/SubSystem176/SubSystem178/SubSystem182/SubSystem236/SubSystem239 ==
  note: Text37
  note: Text36
  Block548 : Inport
  Block549 : Inport  Port=2
  Block550 : Inport  Port=3
  Block551 : Constant  Value=720  OutDataTypeStr=double  SampleTime=-1
  Block552 : Constant  Value=0  OutDataTypeStr=double  SampleTime=-1
  Block553 : Constant  Value=0  OutDataTypeStr=double  SampleTime=-1
  Block554 : Constant  Value=5  OutDataTypeStr=double  SampleTime=-1
  Block555 : DataStoreRead  DataStoreName=DSM50
  Block556 : DataStoreRead  DataStoreName=DSM35
  Block557 : Gain  Gain=5  SaturateOnIntegerOverflow=on  OutDataTypeStr=Inherit: Same as input  ParamDataTypeStr=Inherit: Same as input
      settings: AttributesFormatString=Attribute124
  Block558 : Gain  Gain=5  SaturateOnIntegerOverflow=on  OutDataTypeStr=Inherit: Same as input  ParamDataTypeStr=Inherit: Same as input
      settings: AttributesFormatString=Attribute125
  Block559 : Gain  Gain=1/5  SaturateOnIntegerOverflow=on  OutDataTypeStr=Inherit: Same as input  ParamDataTypeStr=Inherit: Same as input
      settings: AttributesFormatString=Attribute126
  Block560 : Product  Inputs=*/  OutDataTypeStr=double  SaturateOnIntegerOverflow=on  RndMeth=Zero  InputSameDT=on
  Block561 : Rounding
  Block562 : Rounding
  Block563 : Sum  Inputs=+-+-  OutDataTypeStr=double  RndMeth=Zero  SaturateOnIntegerOverflow=on  InputSameDT=on
  Block564 : Sum  Inputs=-+  OutDataTypeStr=double  RndMeth=Zero  SaturateOnIntegerOverflow=on  InputSameDT=on
  SubSystem240 : Subsystem  TreatAsAtomicUnit=on  mask(***ATC.DIVISION***){bd_version=1.01.A, r0=チェック有り, r1=, r2=, r3=, r4=, r5=, r6=, r7=, r8=, r9=, c0=, c1=, c2=, c3=, c4=, c5=, c6=, c7=, c8=, c9=}
      mask prompts: bd_version "Version"
      mask help: これはAutoCからのブロックです．編集禁止．
      settings: OpenFcn=warndlg('変更禁止!');
      mask Help: web(ui_blockhelpmng(gcb));
  SubSystem241 : Subsystem  TreatAsAtomicUnit=on  mask(***ATC.DSRVEC***){bd_version=1.01.A, r0=DSM95, r1=-1, r2=, r3=, r4=, r5=, r6=, r7=, r8=, r9=, c0=, c1=, c2=, c3=, c4=, c5=, c6=, c7=, c8=, c9=}
      mask prompts: bd_version "Version"
      mask help: これはAutoCからのブロックです．編集禁止．
      settings: OpenFcn=warndlg('変更禁止!');
      mask Help: web(ui_blockhelpmng(gcb));
      mask Display: disp('ATC.DSRVEC')
  SubSystem242 : Subsystem
      settings: RTWSystemCode=Inline
  Block573 : Outport
  Block574 : Outport  Port=2
  wires:
  Block562/1 -> Block573/1
  Block559/1 -> Block562/1
  Block558/1 -> Block563/1
  Block555/1 -> Block563/2
  SubSystem241/1(Output) -> SubSystem240/1(mult)
  Block552/1 -> SubSystem240/3(init)
  SubSystem240/1(Out) -> Block563/3  "SigLabel13"
  Block548/1 -> Block558/1
  SubSystem242/1(Block572) -> Block559/1, Block564/2, Block560/1
  Block550/1 -> Block563/4
  Block551/1 -> SubSystem242/1(Block565)
  Block563/1 -> SubSystem242/2(Block566)  "SigLabel12"
  Block553/1 -> SubSystem242/3(Block567)
  Block549/1 -> SubSystem241/1(element)
  Block554/1 -> Block560/2
  Block560/1 -> Block561/1
  Block561/1 -> Block557/1
  Block557/1 -> Block564/1
  Block564/1 -> Block574/1
  Block556/1 -> SubSystem240/2(div)

== system lib_all/SubSystem176/SubSystem178/SubSystem182/SubSystem236/SubSystem245 ==
  note: Text40
  note: Text39
  note: Text38
  Block575 : Inport
  Block576 : Inport  Port=2
  Block577 : Inport  Port=3
  Block578 : Constant  Value=0  OutDataTypeStr=double  SampleTime=-1
  Block579 : Constant  OutDataTypeStr=double  SampleTime=-1
  Block580 : Constant  OutDataTypeStr=double  SampleTime=-1
  Block581 : Constant  Value=0  OutDataTypeStr=double  SampleTime=-1
  Block582 : Constant  Value=2  OutDataTypeStr=double  SampleTime=-1
  Block583 : Constant  OutDataTypeStr=double  SampleTime=-1
  Block584 : Constant  Value=143  OutDataTypeStr=double  SampleTime=-1
  Block585 : Demux  Outputs=2
  Block586 : Logic  Operator=NOT
  Block587 : Mux  Inputs=2
  Block588 : Mux  Inputs=2
  Block589 : Sum  OutDataTypeStr=double  RndMeth=Zero  SaturateOnIntegerOverflow=on  InputSameDT=on
  Block590 : Sum  OutDataTypeStr=double  RndMeth=Zero  SaturateOnIntegerOverflow=on  InputSameDT=on
  Block{591..594} (4 alike) : Switch  Threshold=0.5  RndMeth=Zero  SaturateOnIntegerOverflow=on  InputSameDT=on
  SubSystem246 : Subsystem
  SubSystem247 : Subsystem
  SubSystem248 : Subsystem
  SubSystem249 : Subsystem
  SubSystem250 : Subsystem  TreatAsAtomicUnit=on
  SubSystem259 : Subsystem  TreatAsAtomicUnit=on
  SubSystem269 : Subsystem  TreatAsAtomicUnit=on
  SubSystem279 : Subsystem  TreatAsAtomicUnit=on
  Block654 : Outport
  Block655 : Outport  Port=2
  wires:
  Block593/1 -> Block590/2
  Block583/1 -> Block590/1
  Block575/1 -> SubSystem246/1(In1), Block589/2, Block587/1
  Block584/1 -> SubSystem246/2(In2), Block588/1
  Block576/1 -> SubSystem247/1(In1), SubSystem248/1(In1), SubSystem249/1(In1)
  Block578/1 -> SubSystem247/2(In2)
  SubSystem247/1(Out1) -> Block591/2, SubSystem250/Enable
  SubSystem250/1(Block608) -> Block591/1
  Block579/1 -> SubSystem248/2(In2)
  SubSystem248/1(Out1) -> Block592/2, SubSystem259/Enable
  SubSystem259/1(Block623) -> Block592/1
  Block592/1 -> Block591/3
  Block590/1 -> SubSystem250/1(Block595), SubSystem259/1(Block609), SubSystem269/1(Block624), SubSystem279/1(Block639)
  Block585/1 -> Block654/1
  Block585/2 -> Block655/1
  Block591/1 -> Block585/1
  SubSystem246/1(Out1) -> Block593/2
  Block580/1 -> Block589/1
  Block581/1 -> Block588/2
  Block588/1 -> Block593/3
  Block589/1 -> Block587/2
  Block587/1 -> Block593/1
  Block582/1 -> SubSystem249/2(In2)
  SubSystem249/1(Out1) -> Block586/1, SubSystem269/Enable, Block594/2
  Block586/1 -> SubSystem279/Enable
  SubSystem269/1(Block638) -> Block594/1
  SubSystem279/1(Block653) -> Block594/3
  Block594/1 -> Block592/3
  Block577/1 -> SubSystem250/2(Block596), SubSystem259/2(Block610), SubSystem269/2(Block625), SubSystem279/2(Block640)

== system lib_all/SubSystem176/SubSystem178/SubSystem182/SubSystem289/SubSystem290 ==
  Block662 : Inport
  Block663 : Inport  Port=2
  Block664 : ActionPort
  SubSystem291 : Subsystem  TreatAsAtomicUnit=on  mask(***ATC.DSWVEC***){bd_version=1.01.A, r0=DSM376, r1=-1, r2=, r3=, r4=, r5=, r6=, r7=, r8=, r9=, c0=, c1=, c2=, c3=, c4=, c5=, c6=, c7=, c8=, c9=}
      mask prompts: bd_version "Version"
      mask help: これはAutoCからのブロックです．編集禁止．
      settings: OpenFcn=warndlg('変更禁止!');
      mask Help: web(ui_blockhelpmng(gcb));
      mask Display: disp('ATC.DSRVEC')
  wires:
  Block663/1 -> SubSystem291/2(element)
  Block662/1 -> SubSystem291/1(input)

== system lib_all/SubSystem176/SubSystem178/SubSystem182/SubSystem289/SubSystem292 ==
  Block665 : Inport
  Block666 : Inport  Port=2
  Block667 : ActionPort
  SubSystem293 : Subsystem  TreatAsAtomicUnit=on  mask(***ATC.DSWVEC***){bd_version=1.01.A, r0=DSM397, r1=-1, r2=, r3=, r4=, r5=, r6=, r7=, r8=, r9=, c0=, c1=, c2=, c3=, c4=, c5=, c6=, c7=, c8=, c9=}
      mask prompts: bd_version "Version"
      mask help: これはAutoCからのブロックです．編集禁止．
      settings: OpenFcn=warndlg('変更禁止!');
      mask Help: web(ui_blockhelpmng(gcb));
      mask Display: disp('ATC.DSRVEC')
  wires:
  Block666/1 -> SubSystem293/2(element)
  Block665/1 -> SubSystem293/1(input)

== system lib_all/SubSystem176/SubSystem178/SubSystem182/SubSystem289/SubSystem294 ==
  Block668 : Inport
  Block669 : Inport  Port=2
  Block670 : ActionPort
  SubSystem295 : Subsystem  TreatAsAtomicUnit=on  mask(***ATC.DSWVEC***){bd_version=1.01.A, r0=DSM401, r1=-1, r2=, r3=, r4=, r5=, r6=, r7=, r8=, r9=, c0=, c1=, c2=, c3=, c4=, c5=, c6=, c7=, c8=, c9=}
      mask prompts: bd_version "Version"
      mask help: これはAutoCからのブロックです．編集禁止．
      settings: OpenFcn=warndlg('変更禁止!');
      mask Help: web(ui_blockhelpmng(gcb));
      mask Display: disp('ATC.DSRVEC')
  wires:
  Block669/1 -> SubSystem295/2(element)
  Block668/1 -> SubSystem295/1(input)

== system lib_all/SubSystem176/SubSystem178/SubSystem182/SubSystem296/SubSystem297 ==
  Block676 : Inport
  Block677 : Inport  Port=2
  Block678 : ActionPort
  SubSystem298 : Subsystem  TreatAsAtomicUnit=on  mask(***ATC.DSWVEC***){bd_version=1.01.A, r0=DSM380, r1=-1, r2=, r3=, r4=, r5=, r6=, r7=, r8=, r9=, c0=, c1=, c2=, c3=, c4=, c5=, c6=, c7=, c8=, c9=}
      mask prompts: bd_version "Version"
      mask help: これはAutoCからのブロックです．編集禁止．
      settings: OpenFcn=warndlg('変更禁止!');
      mask Help: web(ui_blockhelpmng(gcb));
      mask Display: disp('ATC.DSRVEC')
  wires:
  Block676/1 -> SubSystem298/1(input)
  Block677/1 -> SubSystem298/2(element)

== system lib_all/SubSystem176/SubSystem178/SubSystem182/SubSystem296/SubSystem299 ==
  Block679 : Inport
  Block680 : Inport  Port=2
  Block681 : ActionPort
  SubSystem300 : Subsystem  TreatAsAtomicUnit=on  mask(***ATC.DSWVEC***){bd_version=1.01.A, r0=DSM398, r1=-1, r2=, r3=, r4=, r5=, r6=, r7=, r8=, r9=, c0=, c1=, c2=, c3=, c4=, c5=, c6=, c7=, c8=, c9=}
      mask prompts: bd_version "Version"
      mask help: これはAutoCからのブロックです．編集禁止．
      settings: OpenFcn=warndlg('変更禁止!');
      mask Help: web(ui_blockhelpmng(gcb));
      mask Display: disp('ATC.DSRVEC')
  wires:
  Block679/1 -> SubSystem300/1(input)
  Block680/1 -> SubSystem300/2(element)

== system lib_all/SubSystem176/SubSystem178/SubSystem182/SubSystem296/SubSystem301 ==
  Block682 : Inport
  Block683 : Inport  Port=2
  Block684 : ActionPort
  SubSystem302 : Subsystem  TreatAsAtomicUnit=on  mask(***ATC.DSWVEC***){bd_version=1.01.A, r0=DSM402, r1=-1, r2=, r3=, r4=, r5=, r6=, r7=, r8=, r9=, c0=, c1=, c2=, c3=, c4=, c5=, c6=, c7=, c8=, c9=}
      mask prompts: bd_version "Version"
      mask help: これはAutoCからのブロックです．編集禁止．
      settings: OpenFcn=warndlg('変更禁止!');
      mask Help: web(ui_blockhelpmng(gcb));
      mask Display: disp('ATC.DSRVEC')
  wires:
  Block682/1 -> SubSystem302/1(input)
  Block683/1 -> SubSystem302/2(element)

== system lib_all/SubSystem176/SubSystem303/SubSystem305/SubSystem308/SubSystem309 ==
  note: ***編集禁止***
  In1 : Inport
  In2 : Inport  Port=2
  RO1 : RelationalOperator  Operator=<=  InputSameDT=on
  single1 : DataTypeConversion  OutDataTypeStr=single  SaturateOnIntegerOverflow=on  RndMeth=Zero
  single2 : DataTypeConversion  OutDataTypeStr=single  SaturateOnIntegerOverflow=on  RndMeth=Zero
  Out1 : Outport
  wires:
  RO1/1 -> Out1/1
  In2/1 -> single2/1
  In1/1 -> single1/1
  single1/1 -> RO1/1
  single2/1 -> RO1/2

== system lib_all/SubSystem176/SubSystem303/SubSystem305/SubSystem308/SubSystem310 ==
  note: ***編集禁止***
  input : Inport
  element : Inport  Port=2
  Assignment : Assignment  IndexOptions=Index vector (port)  Indices=1  OutputSizes=[1 1]
  Inside_DSR : DataStoreRead  DataStoreName=DSM377
  Inside_DSW : DataStoreWrite  DataStoreName=DSM377
  wires:
  element/1 -> Assignment/3
  input/1 -> Assignment/2
  Inside_DSR/1 -> Assignment/1
  Assignment/1 -> Inside_DSW/1

== system lib_all/SubSystem176/SubSystem303/SubSystem305/SubSystem308/SubSystem311 ==
  note: ***編集禁止***
  input : Inport
  element : Inport  Port=2
  Assignment : Assignment  IndexOptions=Index vector (port)  Indices=1  OutputSizes=[1 1]
  Inside_DSR : DataStoreRead  DataStoreName=DSM379
  Inside_DSW : DataStoreWrite  DataStoreName=DSM379
  wires:
  element/1 -> Assignment/3
  input/1 -> Assignment/2
  Inside_DSR/1 -> Assignment/1
  Assignment/1 -> Inside_DSW/1

== system lib_all/SubSystem176/SubSystem303/SubSystem305/SubSystem308/SubSystem312 ==
  note: ***編集禁止***
  input : Inport
  element : Inport  Port=2
  Assignment : Assignment  IndexOptions=Index vector (port)  Indices=1  OutputSizes=[1 1]
  Inside_DSR : DataStoreRead  DataStoreName=DSM381
  Inside_DSW : DataStoreWrite  DataStoreName=DSM381
  wires:
  element/1 -> Assignment/3
  input/1 -> Assignment/2
  Inside_DSR/1 -> Assignment/1
  Assignment/1 -> Inside_DSW/1

== system lib_all/SubSystem176/SubSystem303/SubSystem305/SubSystem308/SubSystem313 ==
  Block705 : EnablePort
  Block706 : Constant  Value=Parameter131  OutDataTypeStr=double  SampleTime=-1
  Block707 : Constant  Value=10  OutDataTypeStr=double  SampleTime=-1
  Block708 : DataStoreWrite  DataStoreName=DSM365
  SubSystem314 : Subsystem  TreatAsAtomicUnit=on
  SubSystem316 : Subsystem  TreatAsAtomicUnit=on
      settings: RTWSystemCode=Inline
  wires:
  Block707/1 -> Block708/1
  Block706/1 -> SubSystem316/1(N)
  SubSystem316/1(action) -> SubSystem314/Trigger
  SubSystem316/2(i) -> SubSystem314/1(Block709)

== system lib_all/SubSystem176/SubSystem303/SubSystem305/SubSystem318/SubSystem319 ==
  note: ***編集禁止***
  In1 : Inport
  In2 : Inport  Port=2
  RO1 : RelationalOperator  Operator===  InputSameDT=on
  single1 : DataTypeConversion  OutDataTypeStr=single  SaturateOnIntegerOverflow=on  RndMeth=Zero
  single2 : DataTypeConversion  OutDataTypeStr=single  SaturateOnIntegerOverflow=on  RndMeth=Zero
  Out1 : Outport
  wires:
  RO1/1 -> Out1/1
  In2/1 -> single2/1
  In1/1 -> single1/1
  single1/1 -> RO1/1
  single2/1 -> RO1/2

== system lib_all/SubSystem176/SubSystem303/SubSystem305/SubSystem318/SubSystem320 ==
  Block725 : Inport
  Block726 : Inport  Port=2
  Block727 : MultiPortSwitch  zeroidx=off  RndMeth=Zero  SaturateOnIntegerOverflow=on  InputSameDT=on
  SubSystem321 : Subsystem  TreatAsAtomicUnit=on  mask(***ATC.DSRVEC***){bd_version=1.01.A, r0=DSM376, r1=-1, r2=, r3=, r4=, r5=, r6=, r7=, r8=, r9=, c0=, c1=, c2=, c3=, c4=, c5=, c6=, c7=, c8=, c9=}
      mask prompts: bd_version "Version"
      mask help: これはAutoCからのブロックです．編集禁止．
      settings: OpenFcn=warndlg('変更禁止!');
      mask Help: web(ui_blockhelpmng(gcb));
      mask Display: disp('ATC.DSRVEC')
  SubSystem322 : Subsystem  TreatAsAtomicUnit=on  mask(***ATC.DSRVEC***){bd_version=1.01.A, r0=DSM397, r1=-1, r2=, r3=, r4=, r5=, r6=, r7=, r8=, r9=, c0=, c1=, c2=, c3=, c4=, c5=, c6=, c7=, c8=, c9=}
      mask prompts: bd_version "Version"
      mask help: これはAutoCからのブロックです．編集禁止．
      settings: OpenFcn=warndlg('変更禁止!');
      mask Help: web(ui_blockhelpmng(gcb));
      mask Display: disp('ATC.DSRVEC')
  SubSystem323 : Subsystem  TreatAsAtomicUnit=on  mask(***ATC.DSRVEC***){bd_version=1.01.A, r0=DSM401, r1=-1, r2=, r3=, r4=, r5=, r6=, r7=, r8=, r9=, c0=, c1=, c2=, c3=, c4=, c5=, c6=, c7=, c8=, c9=}
      mask prompts: bd_version "Version"
      mask help: これはAutoCからのブロックです．編集禁止．
      settings: OpenFcn=warndlg('変更禁止!');
      mask Help: web(ui_blockhelpmng(gcb));
      mask Display: disp('ATC.DSRVEC')
  Block728 : Outport
  wires:
  Block726/1 -> SubSystem323/1(element), SubSystem322/1(element), SubSystem321/1(element)
  SubSystem323/1(Output) -> Block727/4
  SubSystem322/1(Output) -> Block727/3
  SubSystem321/1(Output) -> Block727/2
  Block727/1 -> Block728/1
  Block725/1 -> Block727/1

== system lib_all/SubSystem176/SubSystem303/SubSystem305/SubSystem318/SubSystem324 ==
  Block729 : Inport
  Block730 : Inport  Port=2
  Block731 : MultiPortSwitch  zeroidx=off  RndMeth=Zero  SaturateOnIntegerOverflow=on  InputSameDT=on
  SubSystem325 : Subsystem  TreatAsAtomicUnit=on  mask(***ATC.DSRVEC***){bd_version=1.01.A, r0=DSM380, r1=-1, r2=, r3=, r4=, r5=, r6=, r7=, r8=, r9=, c0=, c1=, c2=, c3=, c4=, c5=, c6=, c7=, c8=, c9=}
      mask prompts: bd_version "Version"
      mask help: これはAutoCからのブロックです．編集禁止．
      settings: OpenFcn=warndlg('変更禁止!');
      mask Help: web(ui_blockhelpmng(gcb));
      mask Display: disp('ATC.DSRVEC')
  SubSystem326 : Subsystem  TreatAsAtomicUnit=on  mask(***ATC.DSRVEC***){bd_version=1.01.A, r0=DSM398, r1=-1, r2=, r3=, r4=, r5=, r6=, r7=, r8=, r9=, c0=, c1=, c2=, c3=, c4=, c5=, c6=, c7=, c8=, c9=}
      mask prompts: bd_version "Version"
      mask help: これはAutoCからのブロックです．編集禁止．
      settings: OpenFcn=warndlg('変更禁止!');
      mask Help: web(ui_blockhelpmng(gcb));
      mask Display: disp('ATC.DSRVEC')
  SubSystem327 : Subsystem  TreatAsAtomicUnit=on  mask(***ATC.DSRVEC***){bd_version=1.01.A, r0=DSM402, r1=-1, r2=, r3=, r4=, r5=, r6=, r7=, r8=, r9=, c0=, c1=, c2=, c3=, c4=, c5=, c6=, c7=, c8=, c9=}
      mask prompts: bd_version "Version"
      mask help: これはAutoCからのブロックです．編集禁止．
      settings: OpenFcn=warndlg('変更禁止!');
      mask Help: web(ui_blockhelpmng(gcb));
      mask Display: disp('ATC.DSRVEC')
  Block732 : Outport
  wires:
  Block730/1 -> SubSystem327/1(element), SubSystem326/1(element), SubSystem325/1(element)
  Block731/1 -> Block732/1
  SubSystem325/1(Output) -> Block731/2
  SubSystem326/1(Output) -> Block731/3
  SubSystem327/1(Output) -> Block731/4
  Block729/1 -> Block731/1

== system lib_all/SubSystem176/SubSystem329/SubSystem332/SubSystem337/SubSystem338 ==
  note: ***編集禁止***
  input : Inport
  element : Inport  Port=2
  Assignment : Assignment  IndexOptions=Index vector (port)  Indices=1  OutputSizes=[1 1]
  Inside_DSR : DataStoreRead  DataStoreName=DSM87
  Inside_DSW : DataStoreWrite  DataStoreName=DSM87
  wires:
  element/1 -> Assignment/3
  input/1 -> Assignment/2
  Inside_DSR/1 -> Assignment/1
  Assignment/1 -> Inside_DSW/1

== system lib_all/SubSystem176/SubSystem329/SubSystem332/SubSystem337/SubSystem339 ==
  note: ***編集禁止***
  element : Inport
  Inside_DSR : DataStoreRead  DataStoreName=DSM379
  Selector : Selector  IndexOptions=Index vector (port)  Indices=[1 3]  OutputSizes=1
  Output : Outport
  wires:
  Selector/1 -> Output/1
  element/1 -> Selector/2
  Inside_DSR/1 -> Selector/1
